simulink model "roach2_fengine"  (Simulink 7.9)
settings: Solver=ode45  StartTime=0.0  StopTime=10.0  RelTol=1e-3
undefined symbols (not defined in the file or in its folder): sfp
libraries: xbsIndex_r4 x3392, casper_library_sources x16, xps_library x13, casper_library_misc x6
(part 1 of 38)
settings Model: Creator=mdexter  LastModifiedBy=peter
settings DebuggingCC: FcnCallInpInsideContextMsg=Use local settings
settings GRTTargetCC: CombineOutputUpdateFcns=off  GRTInterface=on

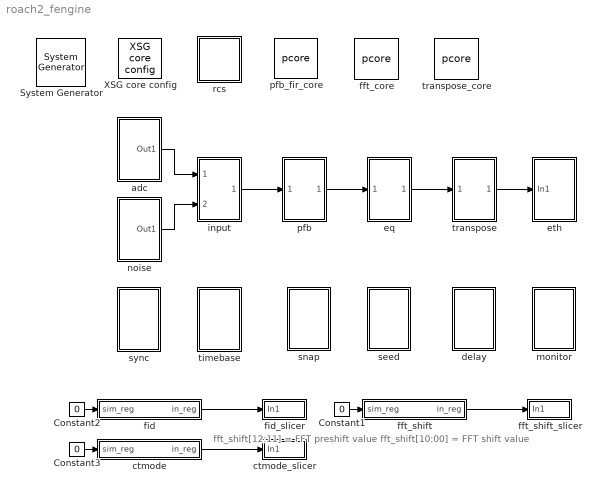
== system roach2_fengine ==
  note: fft_shift[12:11] = FFT preshift value
      | fft_shift[10:00] = FFT shift value
  " System Generator" : xbsIndex_r4/ System Generator  Tag=genX  xilinxfamily=virtex6  part=xc6vsx475t  speed=-1  package=ff1759  synthesis_tool=XST  clock_wrapper=Clock Enables  directory=./roach2_fengine/sysgen  proj_type=Project Navigator  Synth_file=XST Defaults  Impl_file=ISE Defaults  testbench=off  simulink_period=1  sysclk_period=4.1684  dcm_input_clock_period=100  incr_netlist=off  trim_vbits=Everywhere in SubSystem  dbl_ovrd=According to Block Masks  core_generation=According to Block Masks  run_coregen=off  deprecated_control=off  eval_field=0  block_type=sysgen
      settings: block_version=10.1.3
  Constant1 : Constant  Value=0
  Constant2 : Constant  Value=0
  Constant3 : Constant  Value=0
  XSG core config : xps_library/XSG core config  Tag=xps:xsg  hw_sys=ROACH2:sx475t  clk_src=adc0_clk  ROACH_clk_src=adc0_clk  ROACH2_clk_src=adc0_clk  clk_rate=200  sample_period=1  synthesis_tool=XST
  adc : Subsystem
  ctmode : Subsystem  Tag=xps:sw_reg  AncestorBlock=xps_library/software register  mask{numios=1, io_dir=From Processor, io_delay=0, sample_period=1, name1=reg, bitwidth1=32, arith_type1=Unsigned, bin_pt1=0}
      mask prompts: numios "Num I/Os"; io_dir "I/O direction"; io_delay "I/O delay"; sample_period "Sample period"; name1 "Name"; bitwidth1 "Bitwidth"; arith_type1 "Type"; bin_pt1 "Binary pt"
      mask init:
      | swreg_init(gcb);
      mask StyleString: edit,popup(To Processor|From Processor),edit,edit,edit,edit,popup(Unsigned|Signed  (2's comp)|Boolean),edit
      mask CallbackString:
      | t = timer('StartDelay', 0.1); t.TimerFcn = {@swreg_cb, gcb}; start(t);|a = get_param(gcb, 'MaskEnables');
      | if strcmp(get_param(gcb, 'io_dir'), 'To Processor'),
      | a(4) = {'off'};
      | else
      | a(4) = {'on'};
      | end
      | set_param(gcb, 'MaskEnables', a);
      | |||||a = get_param(gcb, 'MaskEnables');
      | if strcmp(get_param(gcb, 'arith_type1'), 'Boolean'),
      | set_param(gcb, 'bitwidth1', '1');
      | set_param(gcb, 'bin_pt1', '0');
      | a(6) = {'off'};
      | a(8) = {'off'};
      | else
      | a(6) = {'on'};
      | a(8) = {'on'};
      | end
      | set_param(gcb, 'MaskEnables', a);
      | |
      mask TabNameString: Setup,Setup,Setup,Setup,IO1,IO1,IO1,IO1
  ctmode_slicer : Subsystem
  delay : Subsystem
  eq : Subsystem
  eth : Subsystem
  fft_core : xps_library/pcore  Tag=xps:pcore  pcore_path=fft_1024ch_core/fft_1024ch_core.ngc
  fft_shift : Subsystem  Tag=xps:sw_reg  AncestorBlock=xps_library/software register  mask{numios=1, io_dir=From Processor, io_delay=0, sample_period=1, name1=reg, bitwidth1=32, arith_type1=Unsigned, bin_pt1=0}
      mask prompts: numios "Num I/Os"; io_dir "I/O direction"; io_delay "I/O delay"; sample_period "Sample period"; name1 "Name"; bitwidth1 "Bitwidth"; arith_type1 "Type"; bin_pt1 "Binary pt"
      mask init:
      | swreg_init(gcb);
      mask StyleString: edit,popup(To Processor|From Processor),edit,edit,edit,edit,popup(Unsigned|Signed  (2's comp)|Boolean),edit
      mask CallbackString:
      | t = timer('StartDelay', 0.1); t.TimerFcn = {@swreg_cb, gcb}; start(t);|a = get_param(gcb, 'MaskEnables');
      | if strcmp(get_param(gcb, 'io_dir'), 'To Processor'),
      | a(4) = {'off'};
      | else
      | a(4) = {'on'};
      | end
      | set_param(gcb, 'MaskEnables', a);
      | |||||a = get_param(gcb, 'MaskEnables');
      | if strcmp(get_param(gcb, 'arith_type1'), 'Boolean'),
      | set_param(gcb, 'bitwidth1', '1');
      | set_param(gcb, 'bin_pt1', '0');
      | a(6) = {'off'};
      | a(8) = {'off'};
      | else
      | a(6) = {'on'};
      | a(8) = {'on'};
      | end
      | set_param(gcb, 'MaskEnables', a);
      | |
      mask TabNameString: Setup,Setup,Setup,Setup,IO1,IO1,IO1,IO1
  fft_shift_slicer : Subsystem
  fid : Subsystem  Tag=xps:sw_reg  AncestorBlock=xps_library/software register  mask{numios=1, io_dir=From Processor, io_delay=0, sample_period=1, name1=reg, bitwidth1=32, arith_type1=Unsigned, bin_pt1=0}
      mask prompts: numios "Num I/Os"; io_dir "I/O direction"; io_delay "I/O delay"; sample_period "Sample period"; name1 "Name"; bitwidth1 "Bitwidth"; arith_type1 "Type"; bin_pt1 "Binary pt"
      mask init:
      | swreg_init(gcb);
      mask StyleString: edit,popup(To Processor|From Processor),edit,edit,edit,edit,popup(Unsigned|Signed  (2's comp)|Boolean),edit
      mask CallbackString:
      | t = timer('StartDelay', 0.1); t.TimerFcn = {@swreg_cb, gcb}; start(t);|a = get_param(gcb, 'MaskEnables');
      | if strcmp(get_param(gcb, 'io_dir'), 'To Processor'),
      | a(4) = {'off'};
      | else
      | a(4) = {'on'};
      | end
      | set_param(gcb, 'MaskEnables', a);
      | |||||a = get_param(gcb, 'MaskEnables');
      | if strcmp(get_param(gcb, 'arith_type1'), 'Boolean'),
      | set_param(gcb, 'bitwidth1', '1');
      | set_param(gcb, 'bin_pt1', '0');
      | a(6) = {'off'};
      | a(8) = {'off'};
      | else
      | a(6) = {'on'};
      | a(8) = {'on'};
      | end
      | set_param(gcb, 'MaskEnables', a);
      | |
      mask TabNameString: Setup,Setup,Setup,Setup,IO1,IO1,IO1,IO1
  fid_slicer : Subsystem
  input : Subsystem
  monitor : Subsystem
  noise : Subsystem
  pfb : Subsystem
  pfb_fir_core : xps_library/pcore  Tag=xps:pcore  pcore_path=pfb_fir_1024ch_core/pfb_fir_1024ch_core.ngc
  rcs : Subsystem  AncestorBlock=casper_library_misc/rcs  mask(rcs){app_src=git, lib_src=git, user_val=0}
      mask prompts: app_src "Application revision info to capture"; lib_src "CASPER libraries revision info to capture"; user_val "User specified value"
      mask help:
      | Embeds revision control information for the user design and libraries.
      | On failure to locate specified revision information, falls back to
      | timestamps (current time for libraries).
      | A user specified value is also stored.
      mask init:
      | rcs_init(gcb, ...
      |     'app_src', app_src, ...
      |     'lib_src', lib_src);
      mask StyleString: popup(timestamp|git|svn),popup(timestamp|git|svn),edit
  seed : Subsystem
  snap : Subsystem
  sync : Subsystem
  timebase : Subsystem
  transpose : Subsystem
  transpose_core : xps_library/pcore  Tag=xps:pcore  pcore_path=transpose_core/transpose_core.ngc
  wires:
  input/1(Out1) -> pfb/1(In1)
  pfb/1(Out1) -> eq/1(In1)
  eq/1(Out2) -> transpose/1(In1)
  adc/1(Out1) -> input/1(In1)
  noise/1(Out1) -> input/2(In2)
  transpose/1(Out1) -> eth/1(In1)
  fft_shift/1(in_reg) -> fft_shift_slicer/1(In1)
  Constant1/1 -> fft_shift/1(sim_reg)
  fid/1(in_reg) -> fid_slicer/1(In1)
  Constant2/1 -> fid/1(sim_reg)
  ctmode/1(in_reg) -> ctmode_slicer/1(In1)
  Constant3/1 -> ctmode/1(sim_reg)

== system roach2_fengine/adc ==
  Bus Creator : BusCreator  Inputs=32
  Constant{3..34} (32 alike) : Constant  Value=0
  adc16x250-8 : Subsystem  Tag=xps:adc16  AncestorBlock=xps_library/ADCs/adc16x250-8  mask(ADC16){board_count=2, roach2_rev=2, zdok_rev=2}
      mask prompts: board_count "Number of ADC16 Boards"; roach2_rev "ROACH2 Revision"; zdok_rev "ZDOK Pinout Revision"
      mask help: 16-input ADC. When using one ADC16 board, it must be in ZDOK0.
      mask init:
      | % Only board_count is needed by init function
      | adc16_init(gcb, ...
      |     'board_count', board_count);
      settings: AttributesFormatString=r2 rev%<roach2_rev>\nzdok rev%<zdok_rev>
      mask Help:
      | This is the CASPER "yellow block" for the CASPER ADC16 board.  Each ADC
      | board has four ADC chips.  Each ADC chip accepts four inputs.  The four
      | 8-bit outputs from each ADC chip are concatenated into a 32-bit signal.
      mask StyleString: popup(1|2),popup(1|2),popup(1|2)
  Out1 : Outport
  wires:
  Constant3/1 -> adc16x250-8/1(a1_sim)
  Constant4/1 -> adc16x250-8/2(a2_sim)
  Constant5/1 -> adc16x250-8/3(a3_sim)
  Constant6/1 -> adc16x250-8/4(a4_sim)
  Constant7/1 -> adc16x250-8/5(b1_sim)
  Constant8/1 -> adc16x250-8/6(b2_sim)
  Constant9/1 -> adc16x250-8/7(b3_sim)
  Constant33/1 -> adc16x250-8/8(b4_sim)
  Constant34/1 -> adc16x250-8/9(c1_sim)
  Constant10/1 -> adc16x250-8/10(c2_sim)
  Constant11/1 -> adc16x250-8/11(c3_sim)
  Constant12/1 -> adc16x250-8/12(c4_sim)
  Constant13/1 -> adc16x250-8/13(d1_sim)
  Constant14/1 -> adc16x250-8/14(d2_sim)
  Constant15/1 -> adc16x250-8/15(d3_sim)
  Constant17/1 -> adc16x250-8/16(d4_sim)
  Constant16/1 -> adc16x250-8/17(e1_sim)
  Constant25/1 -> adc16x250-8/18(e2_sim)
  Constant26/1 -> adc16x250-8/19(e3_sim)
  Constant27/1 -> adc16x250-8/20(e4_sim)
  Constant28/1 -> adc16x250-8/21(f1_sim)
  Constant29/1 -> adc16x250-8/22(f2_sim)
  Constant30/1 -> adc16x250-8/23(f3_sim)
  Constant31/1 -> adc16x250-8/24(f4_sim)
  Constant32/1 -> adc16x250-8/25(g1_sim)
  Constant18/1 -> adc16x250-8/26(g2_sim)
  Constant19/1 -> adc16x250-8/27(g3_sim)
  Constant20/1 -> adc16x250-8/28(g4_sim)
  Constant21/1 -> adc16x250-8/29(h1_sim)
  Constant22/1 -> adc16x250-8/30(h2_sim)
  Constant23/1 -> adc16x250-8/31(h3_sim)
  Constant24/1 -> adc16x250-8/32(h4_sim)
  Bus Creator/1 -> Out1/1
  adc16x250-8/1(a1) -> Bus Creator/1
  adc16x250-8/2(a2) -> Bus Creator/2
  adc16x250-8/3(a3) -> Bus Creator/3
  adc16x250-8/4(a4) -> Bus Creator/4
  adc16x250-8/5(b1) -> Bus Creator/5
  adc16x250-8/6(b2) -> Bus Creator/6
  adc16x250-8/7(b3) -> Bus Creator/7
  adc16x250-8/8(b4) -> Bus Creator/8
  adc16x250-8/9(c1) -> Bus Creator/9
  adc16x250-8/10(c2) -> Bus Creator/10
  adc16x250-8/11(c3) -> Bus Creator/11
  adc16x250-8/12(c4) -> Bus Creator/12
  adc16x250-8/13(d1) -> Bus Creator/13
  adc16x250-8/14(d2) -> Bus Creator/14
  adc16x250-8/15(d3) -> Bus Creator/15
  adc16x250-8/16(d4) -> Bus Creator/16
  adc16x250-8/17(e1) -> Bus Creator/17
  adc16x250-8/18(e2) -> Bus Creator/18
  adc16x250-8/19(e3) -> Bus Creator/19
  adc16x250-8/20(e4) -> Bus Creator/20
  adc16x250-8/21(f1) -> Bus Creator/21
  adc16x250-8/22(f2) -> Bus Creator/22
  adc16x250-8/23(f3) -> Bus Creator/23
  adc16x250-8/24(f4) -> Bus Creator/24
  adc16x250-8/25(g1) -> Bus Creator/25
  adc16x250-8/26(g2) -> Bus Creator/26
  adc16x250-8/27(g3) -> Bus Creator/27
  adc16x250-8/28(g4) -> Bus Creator/28
  adc16x250-8/29(h1) -> Bus Creator/29
  adc16x250-8/30(h2) -> Bus Creator/30
  adc16x250-8/31(h3) -> Bus Creator/31
  adc16x250-8/32(h4) -> Bus Creator/32

== system roach2_fengine/ctmode ==
  sim_reg : Inport
  io_delay : xbsIndex_r4/Delay  rst=off  infoeditControl=Selection of Reset will increase slice count due to use of real FFs and instead of SRLs  en=off  latency=0  dbl_ovrd=off  reg_retiming=on  block_type=delay
  reint1 : xbsIndex_r4/Reinterpret  force_arith_type=on  arith_type=Unsigned  force_bin_pt=on  bin_pt=0  block_type=reinterpret
  roach2_fengine_ctmode_user_data_out : xbsIndex_r4/Gateway In  gui_display_data_type=Fixed-point  arith_type=Unsigned  n_bits=32  bin_pt=0  preci_type=Single  exp_width=8  frac_width=24  quantization="Round  (unbiased: +/- Inf)"  overflow=Saturate  period=1  dbl_ovrd=off  timing_constraint=None  locs_specified=off  LOCs={}  UseAsADC=off  ADCChannel='1'  inherit_from_input=off  hdl_port=on  block_type=gatewayin
  slice_reg : xbsIndex_r4/Slice  nbits=32  boolean_output=off  mode=Lower Bit Location + Width  bit1=0  base1=MSB of Input  bit0=0  base0=LSB of Input  dbl_ovrd=off  block_type=slice
  in_reg : Outport
  wires:
  roach2_fengine_ctmode_user_data_out/1 -> io_delay/1
  sim_reg/1 -> roach2_fengine_ctmode_user_data_out/1
  io_delay/1 -> slice_reg/1
  slice_reg/1 -> reint1/1
  reint1/1 -> in_reg/1

== system roach2_fengine/ctmode_slicer ==
  In1 : Inport
  Delay8 : xbsIndex_r4/Delay  rst=off  infoeditControl=Selection of Reset will increase slice count due to use of real FFs and instead of SRLs  en=off  latency=2  dbl_ovrd=off  reg_retiming=on  block_type=delay
      settings: xl_area=[0, 0, 0, 0, 0, 0, 0]
  b1_b0 : xbsIndex_r4/Slice  nbits=2  boolean_output=off  mode=Lower Bit Location + Width  bit1=0  base1=MSB of Input  bit0=0  base0=LSB of Input  dbl_ovrd=off  block_type=slice
      settings: block_version=10.1.3
  tag scope: ctmode=global
  wires:
  Delay8/1 -> b1_b0/1
  b1_b0/1 -> [ctmode]
  In1/1 -> Delay8/1

== system roach2_fengine/delay ==
  0 : Subsystem  Tag=xps:sw_reg  AncestorBlock=xps_library/software register  mask{numios=1, io_dir=From Processor, io_delay=0, sample_period=1, name1=reg, bitwidth1=32, arith_type1=Unsigned, bin_pt1=0}
      mask prompts: numios "Num I/Os"; io_dir "I/O direction"; io_delay "I/O delay"; sample_period "Sample period"; name1 "Name"; bitwidth1 "Bitwidth"; arith_type1 "Type"; bin_pt1 "Binary pt"
      mask init:
      | swreg_init(gcb);
      mask StyleString: edit,popup(To Processor|From Processor),edit,edit,edit,edit,popup(Unsigned|Signed  (2's comp)|Boolean),edit
      mask CallbackString:
      | t = timer('StartDelay', 0.1); t.TimerFcn = {@swreg_cb, gcb}; start(t);|a = get_param(gcb, 'MaskEnables');
      | if strcmp(get_param(gcb, 'io_dir'), 'To Processor'),
      | a(4) = {'off'};
      | else
      | a(4) = {'on'};
      | end
      | set_param(gcb, 'MaskEnables', a);
      | |||||a = get_param(gcb, 'MaskEnables');
      | if strcmp(get_param(gcb, 'arith_type1'), 'Boolean'),
      | set_param(gcb, 'bitwidth1', '1');
      | set_param(gcb, 'bin_pt1', '0');
      | a(6) = {'off'};
      | a(8) = {'off'};
      | else
      | a(6) = {'on'};
      | a(8) = {'on'};
      | end
      | set_param(gcb, 'MaskEnables', a);
      | |
      mask TabNameString: Setup,Setup,Setup,Setup,IO1,IO1,IO1,IO1
  1 : Subsystem  Tag=xps:sw_reg  AncestorBlock=xps_library/software register  mask{numios=1, io_dir=From Processor, io_delay=0, sample_period=1, name1=reg, bitwidth1=32, arith_type1=Unsigned, bin_pt1=0}
      mask prompts: numios "Num I/Os"; io_dir "I/O direction"; io_delay "I/O delay"; sample_period "Sample period"; name1 "Name"; bitwidth1 "Bitwidth"; arith_type1 "Type"; bin_pt1 "Binary pt"
      mask init:
      | swreg_init(gcb);
      mask StyleString: edit,popup(To Processor|From Processor),edit,edit,edit,edit,popup(Unsigned|Signed  (2's comp)|Boolean),edit
      mask CallbackString:
      | t = timer('StartDelay', 0.1); t.TimerFcn = {@swreg_cb, gcb}; start(t);|a = get_param(gcb, 'MaskEnables');
      | if strcmp(get_param(gcb, 'io_dir'), 'To Processor'),
      | a(4) = {'off'};
      | else
      | a(4) = {'on'};
      | end
      | set_param(gcb, 'MaskEnables', a);
      | |||||a = get_param(gcb, 'MaskEnables');
      | if strcmp(get_param(gcb, 'arith_type1'), 'Boolean'),
      | set_param(gcb, 'bitwidth1', '1');
      | set_param(gcb, 'bin_pt1', '0');
      | a(6) = {'off'};
      | a(8) = {'off'};
      | else
      | a(6) = {'on'};
      | a(8) = {'on'};
      | end
      | set_param(gcb, 'MaskEnables', a);
      | |
      mask TabNameString: Setup,Setup,Setup,Setup,IO1,IO1,IO1,IO1
  2 : Subsystem  Tag=xps:sw_reg  AncestorBlock=xps_library/software register  mask{numios=1, io_dir=From Processor, io_delay=0, sample_period=1, name1=reg, bitwidth1=32, arith_type1=Unsigned, bin_pt1=0}
      mask prompts: numios "Num I/Os"; io_dir "I/O direction"; io_delay "I/O delay"; sample_period "Sample period"; name1 "Name"; bitwidth1 "Bitwidth"; arith_type1 "Type"; bin_pt1 "Binary pt"
      mask init:
      | swreg_init(gcb);
      mask StyleString: edit,popup(To Processor|From Processor),edit,edit,edit,edit,popup(Unsigned|Signed  (2's comp)|Boolean),edit
      mask CallbackString:
      | t = timer('StartDelay', 0.1); t.TimerFcn = {@swreg_cb, gcb}; start(t);|a = get_param(gcb, 'MaskEnables');
      | if strcmp(get_param(gcb, 'io_dir'), 'To Processor'),
      | a(4) = {'off'};
      | else
      | a(4) = {'on'};
      | end
      | set_param(gcb, 'MaskEnables', a);
      | |||||a = get_param(gcb, 'MaskEnables');
      | if strcmp(get_param(gcb, 'arith_type1'), 'Boolean'),
      | set_param(gcb, 'bitwidth1', '1');
      | set_param(gcb, 'bin_pt1', '0');
      | a(6) = {'off'};
      | a(8) = {'off'};
      | else
      | a(6) = {'on'};
      | a(8) = {'on'};
      | end
      | set_param(gcb, 'MaskEnables', a);
      | |
      mask TabNameString: Setup,Setup,Setup,Setup,IO1,IO1,IO1,IO1
  3 : Subsystem  Tag=xps:sw_reg  AncestorBlock=xps_library/software register  mask{numios=1, io_dir=From Processor, io_delay=0, sample_period=1, name1=reg, bitwidth1=32, arith_type1=Unsigned, bin_pt1=0}
      mask prompts: numios "Num I/Os"; io_dir "I/O direction"; io_delay "I/O delay"; sample_period "Sample period"; name1 "Name"; bitwidth1 "Bitwidth"; arith_type1 "Type"; bin_pt1 "Binary pt"
      mask init:
      | swreg_init(gcb);
      mask StyleString: edit,popup(To Processor|From Processor),edit,edit,edit,edit,popup(Unsigned|Signed  (2's comp)|Boolean),edit
      mask CallbackString:
      | t = timer('StartDelay', 0.1); t.TimerFcn = {@swreg_cb, gcb}; start(t);|a = get_param(gcb, 'MaskEnables');
      | if strcmp(get_param(gcb, 'io_dir'), 'To Processor'),
      | a(4) = {'off'};
      | else
      | a(4) = {'on'};
      | end
      | set_param(gcb, 'MaskEnables', a);
      | |||||a = get_param(gcb, 'MaskEnables');
      | if strcmp(get_param(gcb, 'arith_type1'), 'Boolean'),
      | set_param(gcb, 'bitwidth1', '1');
      | set_param(gcb, 'bin_pt1', '0');
      | a(6) = {'off'};
      | a(8) = {'off'};
      | else
      | a(6) = {'on'};
      | a(8) = {'on'};
      | end
      | set_param(gcb, 'MaskEnables', a);
      | |
      mask TabNameString: Setup,Setup,Setup,Setup,IO1,IO1,IO1,IO1
  4 : Subsystem  Tag=xps:sw_reg  AncestorBlock=xps_library/software register  mask{numios=1, io_dir=From Processor, io_delay=0, sample_period=1, name1=reg, bitwidth1=32, arith_type1=Unsigned, bin_pt1=0}
      mask prompts: numios "Num I/Os"; io_dir "I/O direction"; io_delay "I/O delay"; sample_period "Sample period"; name1 "Name"; bitwidth1 "Bitwidth"; arith_type1 "Type"; bin_pt1 "Binary pt"
      mask init:
      | swreg_init(gcb);
      mask StyleString: edit,popup(To Processor|From Processor),edit,edit,edit,edit,popup(Unsigned|Signed  (2's comp)|Boolean),edit
      mask CallbackString:
      | t = timer('StartDelay', 0.1); t.TimerFcn = {@swreg_cb, gcb}; start(t);|a = get_param(gcb, 'MaskEnables');
      | if strcmp(get_param(gcb, 'io_dir'), 'To Processor'),
      | a(4) = {'off'};
      | else
      | a(4) = {'on'};
      | end
      | set_param(gcb, 'MaskEnables', a);
      | |||||a = get_param(gcb, 'MaskEnables');
      | if strcmp(get_param(gcb, 'arith_type1'), 'Boolean'),
      | set_param(gcb, 'bitwidth1', '1');
      | set_param(gcb, 'bin_pt1', '0');
      | a(6) = {'off'};
      | a(8) = {'off'};
      | else
      | a(6) = {'on'};
      | a(8) = {'on'};
      | end
      | set_param(gcb, 'MaskEnables', a);
      | |
      mask TabNameString: Setup,Setup,Setup,Setup,IO1,IO1,IO1,IO1
  5 : Subsystem  Tag=xps:sw_reg  AncestorBlock=xps_library/software register  mask{numios=1, io_dir=From Processor, io_delay=0, sample_period=1, name1=reg, bitwidth1=32, arith_type1=Unsigned, bin_pt1=0}
      mask prompts: numios "Num I/Os"; io_dir "I/O direction"; io_delay "I/O delay"; sample_period "Sample period"; name1 "Name"; bitwidth1 "Bitwidth"; arith_type1 "Type"; bin_pt1 "Binary pt"
      mask init:
      | swreg_init(gcb);
      mask StyleString: edit,popup(To Processor|From Processor),edit,edit,edit,edit,popup(Unsigned|Signed  (2's comp)|Boolean),edit
      mask CallbackString:
      | t = timer('StartDelay', 0.1); t.TimerFcn = {@swreg_cb, gcb}; start(t);|a = get_param(gcb, 'MaskEnables');
      | if strcmp(get_param(gcb, 'io_dir'), 'To Processor'),
      | a(4) = {'off'};
      | else
      | a(4) = {'on'};
      | end
      | set_param(gcb, 'MaskEnables', a);
      | |||||a = get_param(gcb, 'MaskEnables');
      | if strcmp(get_param(gcb, 'arith_type1'), 'Boolean'),
      | set_param(gcb, 'bitwidth1', '1');
      | set_param(gcb, 'bin_pt1', '0');
      | a(6) = {'off'};
      | a(8) = {'off'};
      | else
      | a(6) = {'on'};
      | a(8) = {'on'};
      | end
      | set_param(gcb, 'MaskEnables', a);
      | |
      mask TabNameString: Setup,Setup,Setup,Setup,IO1,IO1,IO1,IO1
  6 : Subsystem  Tag=xps:sw_reg  AncestorBlock=xps_library/software register  mask{numios=1, io_dir=From Processor, io_delay=0, sample_period=1, name1=reg, bitwidth1=32, arith_type1=Unsigned, bin_pt1=0}
      mask prompts: numios "Num I/Os"; io_dir "I/O direction"; io_delay "I/O delay"; sample_period "Sample period"; name1 "Name"; bitwidth1 "Bitwidth"; arith_type1 "Type"; bin_pt1 "Binary pt"
      mask init:
      | swreg_init(gcb);
      mask StyleString: edit,popup(To Processor|From Processor),edit,edit,edit,edit,popup(Unsigned|Signed  (2's comp)|Boolean),edit
      mask CallbackString:
      | t = timer('StartDelay', 0.1); t.TimerFcn = {@swreg_cb, gcb}; start(t);|a = get_param(gcb, 'MaskEnables');
      | if strcmp(get_param(gcb, 'io_dir'), 'To Processor'),
      | a(4) = {'off'};
      | else
      | a(4) = {'on'};
      | end
      | set_param(gcb, 'MaskEnables', a);
      | |||||a = get_param(gcb, 'MaskEnables');
      | if strcmp(get_param(gcb, 'arith_type1'), 'Boolean'),
      | set_param(gcb, 'bitwidth1', '1');
      | set_param(gcb, 'bin_pt1', '0');
      | a(6) = {'off'};
      | a(8) = {'off'};
      | else
      | a(6) = {'on'};
      | a(8) = {'on'};
      | end
      | set_param(gcb, 'MaskEnables', a);
      | |
      mask TabNameString: Setup,Setup,Setup,Setup,IO1,IO1,IO1,IO1
  7 : Subsystem  Tag=xps:sw_reg  AncestorBlock=xps_library/software register  mask{numios=1, io_dir=From Processor, io_delay=0, sample_period=1, name1=reg, bitwidth1=32, arith_type1=Unsigned, bin_pt1=0}
      mask prompts: numios "Num I/Os"; io_dir "I/O direction"; io_delay "I/O delay"; sample_period "Sample period"; name1 "Name"; bitwidth1 "Bitwidth"; arith_type1 "Type"; bin_pt1 "Binary pt"
      mask init:
      | swreg_init(gcb);
      mask StyleString: edit,popup(To Processor|From Processor),edit,edit,edit,edit,popup(Unsigned|Signed  (2's comp)|Boolean),edit
      mask CallbackString:
      | t = timer('StartDelay', 0.1); t.TimerFcn = {@swreg_cb, gcb}; start(t);|a = get_param(gcb, 'MaskEnables');
      | if strcmp(get_param(gcb, 'io_dir'), 'To Processor'),
      | a(4) = {'off'};
      | else
      | a(4) = {'on'};
      | end
      | set_param(gcb, 'MaskEnables', a);
      | |||||a = get_param(gcb, 'MaskEnables');
      | if strcmp(get_param(gcb, 'arith_type1'), 'Boolean'),
      | set_param(gcb, 'bitwidth1', '1');
      | set_param(gcb, 'bin_pt1', '0');
      | a(6) = {'off'};
      | a(8) = {'off'};
      | else
      | a(6) = {'on'};
      | a(8) = {'on'};
      | end
      | set_param(gcb, 'MaskEnables', a);
      | |
      mask TabNameString: Setup,Setup,Setup,Setup,IO1,IO1,IO1,IO1
  BitBasher : xbsIndex_r4/BitBasher  bitexpr=|  display_expr=off  sr_1=1  arith_type1=Unsigned  bin_pt1=0  sr_2=2  arith_type2=Unsigned  bin_pt2=0  sr_3=3  arith_type3=Unsigned  bin_pt3=0  sr_4=4  arith_type4=Unsigned  bin_pt4=0  dbl_ovrd=off  block_type=bitbasher
      | <!DOCTYPE HTML PUBLIC "-//W3C//DTD HTML 4.0//EN" "http://www.w3.org/TR/REC-html40/strict.dtd">
      | <html><head><meta name="qrichtext" content="1" /><style type="text/css">
      | p, li { white-space: pre-wrap; }
      | </style></head><body style=" font-family:'Sans'; font-size:10pt; font-weight:400; font-style:normal;">
      | <p style=" margin-top:12px; margin-bottom:12px; margin-left:0px; margin-right:0px; -qt-block-indent:0; text-indent:0px;"><span style=" font-family:'MS Shell Dlg'; font-size:8pt;">d={b,a}</span></p></body></html>
  BitBasher1 : xbsIndex_r4/BitBasher  bitexpr=|  display_expr=off  sr_1=1  arith_type1=Unsigned  bin_pt1=0  sr_2=2  arith_type2=Unsigned  bin_pt2=0  sr_3=3  arith_type3=Unsigned  bin_pt3=0  sr_4=4  arith_type4=Unsigned  bin_pt4=0  dbl_ovrd=off  block_type=bitbasher
      | <!DOCTYPE HTML PUBLIC "-//W3C//DTD HTML 4.0//EN" "http://www.w3.org/TR/REC-html40/strict.dtd">
      | <html><head><meta name="qrichtext" content="1" /><style type="text/css">
      | p, li { white-space: pre-wrap; }
      | </style></head><body style=" font-family:'Sans'; font-size:10pt; font-weight:400; font-style:normal;">
      | <p style=" margin-top:12px; margin-bottom:12px; margin-left:0px; margin-right:0px; -qt-block-indent:0; text-indent:0px;"><span style=" font-family:'MS Shell Dlg'; font-size:8pt;">d={b,a}</span></p></body></html>
  BitBasher2 : xbsIndex_r4/BitBasher  bitexpr=|  display_expr=off  sr_1=1  arith_type1=Unsigned  bin_pt1=0  sr_2=2  arith_type2=Unsigned  bin_pt2=0  sr_3=3  arith_type3=Unsigned  bin_pt3=0  sr_4=4  arith_type4=Unsigned  bin_pt4=0  dbl_ovrd=off  block_type=bitbasher
      | <!DOCTYPE HTML PUBLIC "-//W3C//DTD HTML 4.0//EN" "http://www.w3.org/TR/REC-html40/strict.dtd">
      | <html><head><meta name="qrichtext" content="1" /><style type="text/css">
      | p, li { white-space: pre-wrap; }
      | </style></head><body style=" font-family:'Sans'; font-size:10pt; font-weight:400; font-style:normal;">
      | <p style=" margin-top:12px; margin-bottom:12px; margin-left:0px; margin-right:0px; -qt-block-indent:0; text-indent:0px;"><span style=" font-family:'MS Shell Dlg'; font-size:8pt;">d={b,a}</span></p></body></html>
  BitBasher3 : xbsIndex_r4/BitBasher  bitexpr=|  display_expr=off  sr_1=1  arith_type1=Unsigned  bin_pt1=0  sr_2=2  arith_type2=Unsigned  bin_pt2=0  sr_3=3  arith_type3=Unsigned  bin_pt3=0  sr_4=4  arith_type4=Unsigned  bin_pt4=0  dbl_ovrd=off  block_type=bitbasher
      | <!DOCTYPE HTML PUBLIC "-//W3C//DTD HTML 4.0//EN" "http://www.w3.org/TR/REC-html40/strict.dtd">
      | <html><head><meta name="qrichtext" content="1" /><style type="text/css">
      | p, li { white-space: pre-wrap; }
      | </style></head><body style=" font-family:'Sans'; font-size:10pt; font-weight:400; font-style:normal;">
      | <p style=" margin-top:12px; margin-bottom:12px; margin-left:0px; margin-right:0px; -qt-block-indent:0; text-indent:0px;"><span style=" font-family:'MS Shell Dlg'; font-size:8pt;">d={b,a}</span></p></body></html>
  Constant{,1..7} (8 alike) : Constant  Value=0
  tag scope: delay0=global, delay1=global, delay2=global, delay3=global
  wires:
  0/1(in_reg) -> BitBasher/1
  Constant/1 -> 0/1(sim_reg)
  1/1(in_reg) -> BitBasher/2
  Constant1/1 -> 1/1(sim_reg)
  2/1(in_reg) -> BitBasher1/1
  Constant2/1 -> 2/1(sim_reg)
  3/1(in_reg) -> BitBasher1/2
  Constant3/1 -> 3/1(sim_reg)
  4/1(in_reg) -> BitBasher2/1
  Constant4/1 -> 4/1(sim_reg)
  5/1(in_reg) -> BitBasher2/2
  Constant5/1 -> 5/1(sim_reg)
  6/1(in_reg) -> BitBasher3/1
  Constant6/1 -> 6/1(sim_reg)
  7/1(in_reg) -> BitBasher3/2
  Constant7/1 -> 7/1(sim_reg)
  BitBasher/1 -> [delay0]
  BitBasher1/1 -> [delay1]
  BitBasher2/1 -> [delay2]
  BitBasher3/1 -> [delay3]

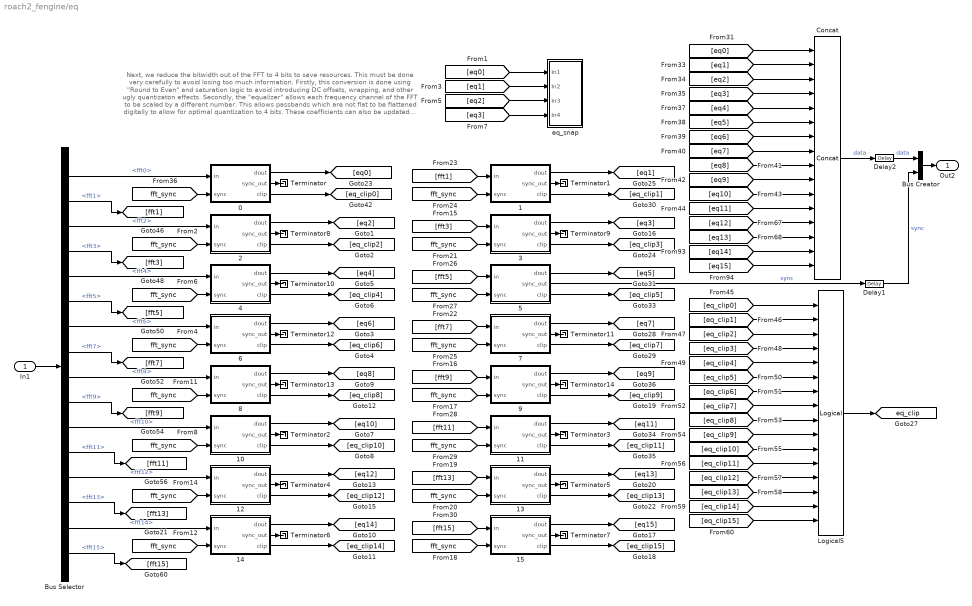
== system roach2_fengine/eq ==
  note: Next, we reduce the bitwidth out of the FFT to 4 bits to save resources.
      | This must be done very carefully to avoid losing too much information.
      | Firstly, this conversion is done using "Round to Even" and saturation
      | logic to avoid introducing DC offsets, wrapping, and other ugly quantizaton
      | effects.  Secondly, the "equalizer" allows each frequency channel of the
      | FFT to be scaled by a different number.  This allows passbands which are
      | not flat to be flattened digitally to allow for optimal quantization to 4 bits.
      | These coefficients can also be updated dynamically for gain control.
      | Coefficients can be dynamically written into the "equalizer" by
      | specifying the channel ("coeff_addr") and the value of the
      | coefficient ("coeff"), and then using "coeff_en" to write the
      | new coefficient into the equalization table.
  In1 : Inport
  0 : Subsystem  mask{nchan=11}
      mask prompts: nchan "Number of fft channels (same as fft prompt) (2^?)"
  1 : Subsystem  mask{nchan=11}
      mask prompts: nchan "Number of fft channels (same as fft prompt) (2^?)"
  10 : Subsystem  mask{nchan=11}
      mask prompts: nchan "Number of fft channels (same as fft prompt) (2^?)"
  11 : Subsystem  mask{nchan=11}
      mask prompts: nchan "Number of fft channels (same as fft prompt) (2^?)"
  12 : Subsystem  mask{nchan=11}
      mask prompts: nchan "Number of fft channels (same as fft prompt) (2^?)"
  13 : Subsystem  mask{nchan=11}
      mask prompts: nchan "Number of fft channels (same as fft prompt) (2^?)"
  14 : Subsystem  mask{nchan=11}
      mask prompts: nchan "Number of fft channels (same as fft prompt) (2^?)"
  15 : Subsystem  mask{nchan=11}
      mask prompts: nchan "Number of fft channels (same as fft prompt) (2^?)"
  2 : Subsystem  mask{nchan=11}
      mask prompts: nchan "Number of fft channels (same as fft prompt) (2^?)"
  3 : Subsystem  mask{nchan=11}
      mask prompts: nchan "Number of fft channels (same as fft prompt) (2^?)"
  4 : Subsystem  mask{nchan=11}
      mask prompts: nchan "Number of fft channels (same as fft prompt) (2^?)"
  5 : Subsystem  mask{nchan=11}
      mask prompts: nchan "Number of fft channels (same as fft prompt) (2^?)"
  6 : Subsystem  mask{nchan=11}
      mask prompts: nchan "Number of fft channels (same as fft prompt) (2^?)"
  7 : Subsystem  mask{nchan=11}
      mask prompts: nchan "Number of fft channels (same as fft prompt) (2^?)"
  8 : Subsystem  mask{nchan=11}
      mask prompts: nchan "Number of fft channels (same as fft prompt) (2^?)"
  9 : Subsystem  mask{nchan=11}
      mask prompts: nchan "Number of fft channels (same as fft prompt) (2^?)"
  Bus Creator : BusCreator  Inputs=2
  Bus Selector : BusSelector  OutputSignals=fft0,fft1,fft2,fft3,fft4,fft5,fft6,fft7,fft8,fft9,fft10,fft11,fft12,fft13,fft14,fft15
  Concat : xbsIndex_r4/Concat  num_inputs=16  dbl_ovrd=off  block_type=concat
  Delay1 : xbsIndex_r4/Delay  rst=off  infoeditControl=Selection of Reset will increase slice count due to use of real FFs and instead of SRLs  en=off  latency=1  dbl_ovrd=off  reg_retiming=off  block_type=delay
  Delay2 : xbsIndex_r4/Delay  rst=off  infoeditControl=Selection of Reset will increase slice count due to use of real FFs and instead of SRLs  en=off  latency=1  dbl_ovrd=off  reg_retiming=off  block_type=delay
  Logical5 : xbsIndex_r4/Logical  logical_function=OR  inputs=16  en=off  latency=1  precision=Full  arith_type=Unsigned  n_bits=8  bin_pt=2  align_bp=on  dbl_ovrd=off  block_type=logical
      settings: xl_area=[0, 0, 0, 0, 0, 0, 0]
  Terminator{,1..14} (15 alike) : Terminator
  eq_snap : Subsystem
  Out2 : Outport
  tag scope: eq_clip=global
  wires:
  0/1(dout) -> [eq0]
  0/3(clip) -> [eq_clip0]
  Logical5/1 -> [eq_clip]
  [fft_sync] -> 0/2(sync)
  Delay2/1 -> Bus Creator/1  "data"
  Bus Creator/1 -> Out2/1
  2/1(dout) -> [eq2]
  2/3(clip) -> [eq_clip2]
  [fft_sync] -> 2/2(sync)
  4/1(dout) -> [eq4]
  Bus Selector/5 -> 4/1(in)  "<fft4>"
  4/3(clip) -> [eq_clip4]
  [fft_sync] -> 4/2(sync)
  6/1(dout) -> [eq6]
  Bus Selector/7 -> 6/1(in)  "<fft6>"
  6/3(clip) -> [eq_clip6]
  [fft_sync] -> 6/2(sync)
  8/1(dout) -> [eq8]
  Bus Selector/9 -> 8/1(in)  "<fft8>"
  8/3(clip) -> [eq_clip8]
  [fft_sync] -> 8/2(sync)
  10/1(dout) -> [eq10]
  Bus Selector/11 -> 10/1(in)  "<fft10>"
  10/3(clip) -> [eq_clip10]
  [fft_sync] -> 10/2(sync)
  12/1(dout) -> [eq12]
  Bus Selector/13 -> 12/1(in)  "<fft12>"
  12/3(clip) -> [eq_clip12]
  [fft_sync] -> 12/2(sync)
  14/1(dout) -> [eq14]
  Bus Selector/15 -> 14/1(in)  "<fft14>"
  14/3(clip) -> [eq_clip14]
  [fft_sync] -> 14/2(sync)
  1/1(dout) -> [eq1]
  1/3(clip) -> [eq_clip1]
  [fft_sync] -> 1/2(sync)
  3/1(dout) -> [eq3]
  [fft3] -> 3/1(in)
  3/3(clip) -> [eq_clip3]
  [fft_sync] -> 3/2(sync)
  5/1(dout) -> [eq5]
  [fft5] -> 5/1(in)
  5/3(clip) -> [eq_clip5]
  [fft_sync] -> 5/2(sync)
  7/1(dout) -> [eq7]
  [fft7] -> 7/1(in)
  7/3(clip) -> [eq_clip7]
  [fft_sync] -> 7/2(sync)
  9/1(dout) -> [eq9]
  [fft9] -> 9/1(in)
  9/3(clip) -> [eq_clip9]
  [fft_sync] -> 9/2(sync)
  11/1(dout) -> [eq11]
  [fft11] -> 11/1(in)
  11/3(clip) -> [eq_clip11]
  [fft_sync] -> 11/2(sync)
  13/1(dout) -> [eq13]
  [fft13] -> 13/1(in)
  13/3(clip) -> [eq_clip13]
  [fft_sync] -> 13/2(sync)
  15/1(dout) -> [eq15]
  [fft15] -> 15/1(in)
  15/3(clip) -> [eq_clip15]
  [fft_sync] -> 15/2(sync)
  [eq0] -> Concat/1
  [eq1] -> Concat/2
  [eq2] -> Concat/3
  [eq3] -> Concat/4
  [eq4] -> Concat/5
  [eq5] -> Concat/6
  [eq6] -> Concat/7
  [eq7] -> Concat/8
  [eq8] -> Concat/9
  [eq9] -> Concat/10
  [eq10] -> Concat/11
  [eq11] -> Concat/12
  [eq12] -> Concat/13
  [eq13] -> Concat/14
  [eq14] -> Concat/15
  [eq15] -> Concat/16
  [eq_clip0] -> Logical5/1
  [eq_clip1] -> Logical5/2
  [eq_clip2] -> Logical5/3
  [eq_clip3] -> Logical5/4
  [eq_clip4] -> Logical5/5
  [eq_clip5] -> Logical5/6
  [eq_clip6] -> Logical5/7
  [eq_clip7] -> Logical5/8
  [eq_clip8] -> Logical5/9
  [eq_clip9] -> Logical5/10
  [eq_clip10] -> Logical5/11
  [eq_clip11] -> Logical5/12
  [eq_clip12] -> Logical5/13
  [eq_clip13] -> Logical5/14
  [eq_clip14] -> Logical5/15
  [eq_clip15] -> Logical5/16
  In1/1 -> Bus Selector/1
  Bus Selector/1 -> 0/1(in)  "<fft0>"
  Bus Selector/3 -> 2/1(in)  "<fft2>"
  [fft1] -> 1/1(in)
  Bus Selector/16 -> [fft15]  "<fft15>"
  Bus Selector/14 -> [fft13]  "<fft13>"
  Bus Selector/12 -> [fft11]  "<fft11>"
  Bus Selector/10 -> [fft9]  "<fft9>"
  Bus Selector/8 -> [fft7]  "<fft7>"
  Bus Selector/6 -> [fft5]  "<fft5>"
  Bus Selector/4 -> [fft3]  "<fft3>"
  Bus Selector/2 -> [fft1]  "<fft1>"
  0/2(sync_out) -> Terminator/1
  1/2(sync_out) -> Terminator1/1
  10/2(sync_out) -> Terminator2/1
  11/2(sync_out) -> Terminator3/1
  12/2(sync_out) -> Terminator4/1
  13/2(sync_out) -> Terminator5/1
  14/2(sync_out) -> Terminator6/1
  15/2(sync_out) -> Terminator7/1
  2/2(sync_out) -> Terminator8/1
  3/2(sync_out) -> Terminator9/1
  4/2(sync_out) -> Terminator10/1
  5/2(sync_out) -> Delay1/1  "sync"
  6/2(sync_out) -> Terminator12/1
  8/2(sync_out) -> Terminator13/1
  9/2(sync_out) -> Terminator14/1
  7/2(sync_out) -> Terminator11/1
  Concat/1 -> Delay2/1  "data"
  Delay1/1 -> Bus Creator/2  "sync"
  [eq0] -> eq_snap/1(In1)
  [eq1] -> eq_snap/2(In2)
  [eq2] -> eq_snap/3(In3)
  [eq3] -> eq_snap/4(In4)

== system roach2_fengine/eth ==
  note: (old)
      | IP and MAC address assignments for ports connected to switch
      | eth0: IP=10.10.10.(32+FID)  MAC=02:02:0A:0A:0A:(20+FID)
      | eth1: IP=10.10.10.(40+FID)  MAC=02:02:0A:0A:0A:(28+FID)
      | eth2: IP=10.10.10.(48+FID)  MAC=02:02:0A:0A:0A:(30+FID)
      | eth3: IP=10.10.10.(56+FID)  MAC=02:02:0A:0A:0A:(38+FID)
  note: ********old********
      | IP and MAC address assignments for ports connected to GPU X engines
      | eth0: IP=192.168.2.(1+FID)  MAC=02:02:C0:A8:02:(1+FID)
      | eth1: IP=192.168.3.(1+FID)  MAC=02:02:C0:A8:03:(1+FID)
      | eth2: IP=192.168.4.(1+FID)  MAC=02:02:C0:A8:04:(1+FID)
      | eth3: IP=192.168.5.(1+FID)  MAC=02:02:C0:A8:05:(1+FID)
  note: ctrl[8] = cnt_rst
      | ctrl[5] = gbe_gpu_rst
      | ctrl[4] = gbe_sw_rdst
      | ctrl[1] = gbe_gpu_en
      | ctrl[0] = gbe_sw_en
  note: (present)
      | IP and MAC address assignments for ports connected to switch
      | eth0: IP=10.10.10.(FID)  MAC=02:02:0A:0A:0A:(FID)
      | eth1: IP=10.10.10.(8+FID)  MAC=02:02:0A:0A:0A:(8+FID)
      | eth2: IP=10.10.10.(16+FID)  MAC=02:02:0A:0A:0A:(10+FID)
      | eth3: IP=10.10.10.(24+FID)  MAC=02:02:0A:0A:0A:(18+FID)
  In1 : Inport
  0 : Subsystem
  1 : Subsystem
  2 : Subsystem
  3 : Subsystem
  BitBasher : xbsIndex_r4/BitBasher  bitexpr=|  display_expr=off  sr_1=1  arith_type1=Unsigned  bin_pt1=0  sr_2=2  arith_type2=Unsigned  bin_pt2=0  sr_3=3  arith_type3=Unsigned  bin_pt3=0  sr_4=4  arith_type4=Unsigned  bin_pt4=0  dbl_ovrd=off  block_type=bitbasher
      | <!DOCTYPE HTML PUBLIC "-//W3C//DTD HTML 4.0//EN" "http://www.w3.org/TR/REC-html40/strict.dtd">
      | <html><head><meta name="qrichtext" content="1" /><style type="text/css">
      | p, li { white-space: pre-wrap; }
      | </style></head><body style=" font-family:'Sans'; font-size:10pt; font-weight:400; font-style:normal;">
      | <p style=" margin-top:12px; margin-bottom:12px; margin-left:0px; margin-right:0px; -qt-block-indent:0; text-indent:0px;"><span style=" font-family:'MS Shell Dlg'; font-size:8pt;">gpurst={ctrl[5]}<br />swrst={ctrl[4]}<br />gpuen={ctrl[1]}<br />swen={ctrl[0]}</span></p></body></html>
  BitBasher1 : xbsIndex_r4/BitBasher  bitexpr=|  display_expr=off  sr_1=1  arith_type1=Unsigned  bin_pt1=0  sr_2=2  arith_type2=Unsigned  bin_pt2=0  sr_3=3  arith_type3=Unsigned  bin_pt3=0  sr_4=4  arith_type4=Unsigned  bin_pt4=0  dbl_ovrd=off  block_type=bitbasher
      | <!DOCTYPE HTML PUBLIC "-//W3C//DTD HTML 4.0//EN" "http://www.w3.org/TR/REC-html40/strict.dtd">
      | <html><head><meta name="qrichtext" content="1" /><style type="text/css">
      | p, li { white-space: pre-wrap; }
      | </style></head><body style=" font-family:'Sans'; font-size:10pt; font-weight:400; font-style:normal;">
      | <p style=" margin-top:12px; margin-bottom:12px; margin-left:0px; margin-right:0px; -qt-block-indent:0; text-indent:0px;"><span style=" font-family:'MS Shell Dlg'; font-size:8pt;">cntrst={ctrl[8]}<br /></span></p></body></html>
  Bus Selector2 : BusSelector  OutputSignals=chan_0_255,chan_256_511,chan_512_767,chan_768_1023
  Constant1 : Constant  Value=0
  Constant3 : xbsIndex_r4/Constant  const=0  gui_display_data_type=Fixed-point  arith_type=Unsigned  n_bits=2  bin_pt=0  preci_type=Single  exp_width=8  frac_width=24  explicit_period=on  period=1  equ=P=C  opselect=C  inp2=PCIN>>17  opr=+  inp1=P  carry=CIN  dbl_ovrd=off  block_type=constant
  Constant5 : xbsIndex_r4/Constant  const=1  gui_display_data_type=Fixed-point  arith_type=Unsigned  n_bits=2  bin_pt=0  preci_type=Single  exp_width=8  frac_width=24  explicit_period=on  period=1  equ=P=C  opselect=C  inp2=PCIN>>17  opr=+  inp1=P  carry=CIN  dbl_ovrd=off  block_type=constant
  Constant7 : xbsIndex_r4/Constant  const=2  gui_display_data_type=Fixed-point  arith_type=Unsigned  n_bits=2  bin_pt=0  preci_type=Single  exp_width=8  frac_width=24  explicit_period=on  period=1  equ=P=C  opselect=C  inp2=PCIN>>17  opr=+  inp1=P  carry=CIN  dbl_ovrd=off  block_type=constant
  Constant8 : xbsIndex_r4/Constant  const=3  gui_display_data_type=Fixed-point  arith_type=Unsigned  n_bits=2  bin_pt=0  preci_type=Single  exp_width=8  frac_width=24  explicit_period=on  period=1  equ=P=C  opselect=C  inp2=PCIN>>17  opr=+  inp1=P  carry=CIN  dbl_ovrd=off  block_type=constant
  Convert{1..5} (5 alike) : xbsIndex_r4/Convert  gui_display_data_type=Boolean  arith_type=Boolean  n_bits=32  bin_pt=0  float_type=Single  exp_bits=8  fraction_bits=24  quantization=Truncate  overflow=Wrap  en=off  latency=0  dbl_ovrd=off  pipeline=off  block_type=convert
      settings: block_version=11.4
  Delay2 : xbsIndex_r4/Delay  rst=off  infoeditControl=Selection of Reset will increase slice count due to use of real FFs and instead of SRLs  en=off  latency=2  dbl_ovrd=off  reg_retiming=on  block_type=delay
  ctrl : Subsystem  Tag=xps:sw_reg  AncestorBlock=xps_library/software register  mask{numios=1, io_dir=From Processor, io_delay=0, sample_period=1, name1=reg, bitwidth1=32, arith_type1=Unsigned, bin_pt1=0}
      mask prompts: numios "Num I/Os"; io_dir "I/O direction"; io_delay "I/O delay"; sample_period "Sample period"; name1 "Name"; bitwidth1 "Bitwidth"; arith_type1 "Type"; bin_pt1 "Binary pt"
      mask init:
      | swreg_init(gcb);
      mask StyleString: edit,popup(To Processor|From Processor),edit,edit,edit,edit,popup(Unsigned|Signed  (2's comp)|Boolean),edit
      mask CallbackString:
      | t = timer('StartDelay', 0.1); t.TimerFcn = {@swreg_cb, gcb}; start(t);|a = get_param(gcb, 'MaskEnables');
      | if strcmp(get_param(gcb, 'io_dir'), 'To Processor'),
      | a(4) = {'off'};
      | else
      | a(4) = {'on'};
      | end
      | set_param(gcb, 'MaskEnables', a);
      | |||||a = get_param(gcb, 'MaskEnables');
      | if strcmp(get_param(gcb, 'arith_type1'), 'Boolean'),
      | set_param(gcb, 'bitwidth1', '1');
      | set_param(gcb, 'bin_pt1', '0');
      | a(6) = {'off'};
      | a(8) = {'off'};
      | else
      | a(6) = {'on'};
      | a(8) = {'on'};
      | end
      | set_param(gcb, 'MaskEnables', a);
      | |
      mask TabNameString: Setup,Setup,Setup,Setup,IO1,IO1,IO1,IO1
  tag scope: cnt_rst=global, gbe_gpu_en=global, gbe_gpu_rst=global, gbe_sw_en=global, gbe_sw_rst=global
  wires:
  In1/1 -> Bus Selector2/1
  [chan_256_511] -> 1/1(data)
  Bus Selector2/1 -> [chan_0_255]  "<chan_0_255>"
  Bus Selector2/2 -> [chan_256_511]  "<chan_256_511>"
  Bus Selector2/3 -> [chan_512_767]  "<chan_512_767>"
  Bus Selector2/4 -> [chan_768_1023]  "<chan_768_1023>"
  [chan_0_255] -> 0/1(data)
  [chan_768_1023] -> 3/1(data)
  [chan_512_767] -> 2/1(data)
  Constant1/1 -> ctrl/1(sim_reg)
  BitBasher/1 -> Convert2/1
  BitBasher/2 -> Convert3/1
  BitBasher/3 -> Convert4/1
  BitBasher/4 -> Convert5/1
  BitBasher1/1 -> Convert1/1
  Convert2/1 -> [gbe_gpu_rst]
  Convert1/1 -> [cnt_rst]
  Convert3/1 -> [gbe_sw_rst]
  Convert4/1 -> [gbe_gpu_en]
  ctrl/1(in_reg) -> Delay2/1
  Delay2/1 -> BitBasher/1, BitBasher1/1
  Constant3/1 -> 0/2(sw_base)
  Constant7/1 -> 2/2(sw_base)
  Constant8/1 -> 3/2(sw_base)
  Constant5/1 -> 1/2(sw_base)
  Convert5/1 -> [gbe_sw_en]

== system roach2_fengine/fft_shift ==
  sim_reg : Inport
  io_delay : xbsIndex_r4/Delay  rst=off  infoeditControl=Selection of Reset will increase slice count due to use of real FFs and instead of SRLs  en=off  latency=0  dbl_ovrd=off  reg_retiming=on  block_type=delay
  reint1 : xbsIndex_r4/Reinterpret  force_arith_type=on  arith_type=Unsigned  force_bin_pt=on  bin_pt=0  block_type=reinterpret
  roach2_fengine_fft_shift_user_data_out : xbsIndex_r4/Gateway In  gui_display_data_type=Fixed-point  arith_type=Unsigned  n_bits=32  bin_pt=0  preci_type=Single  exp_width=8  frac_width=24  quantization="Round  (unbiased: +/- Inf)"  overflow=Saturate  period=1  dbl_ovrd=off  timing_constraint=None  locs_specified=off  LOCs={}  UseAsADC=off  ADCChannel='1'  inherit_from_input=off  hdl_port=on  block_type=gatewayin
  slice_reg : xbsIndex_r4/Slice  nbits=32  boolean_output=off  mode=Lower Bit Location + Width  bit1=0  base1=MSB of Input  bit0=0  base0=LSB of Input  dbl_ovrd=off  block_type=slice
  in_reg : Outport
  wires:
  roach2_fengine_fft_shift_user_data_out/1 -> io_delay/1
  sim_reg/1 -> roach2_fengine_fft_shift_user_data_out/1
  io_delay/1 -> slice_reg/1
  slice_reg/1 -> reint1/1
  reint1/1 -> in_reg/1

== system roach2_fengine/fft_shift_slicer ==
  In1 : Inport
  Convert : xbsIndex_r4/Convert  gui_display_data_type=Fixed-point  arith_type=Unsigned  n_bits=16  bin_pt=0  float_type=Single  exp_bits=8  fraction_bits=24  quantization=Truncate  overflow=Wrap  en=off  latency=0  dbl_ovrd=off  pipeline=off  block_type=convert
  Delay8 : xbsIndex_r4/Delay  rst=off  infoeditControl=Selection of Reset will increase slice count due to use of real FFs and instead of SRLs  en=off  latency=2  dbl_ovrd=off  reg_retiming=on  block_type=delay
      settings: xl_area=[0, 0, 0, 0, 0, 0, 0]
  b10_b0 : xbsIndex_r4/Slice  nbits=11  boolean_output=off  mode=Lower Bit Location + Width  bit1=0  base1=MSB of Input  bit0=0  base0=LSB of Input  dbl_ovrd=off  block_type=slice
      settings: block_version=10.1.3
  b12_b11 : xbsIndex_r4/Slice  nbits=2  boolean_output=off  mode=Lower Bit Location + Width  bit1=0  base1=MSB of Input  bit0=11  base0=LSB of Input  dbl_ovrd=off  block_type=slice
      settings: block_version=10.1.3
  tag scope: fft_preshift=global, fft_shift=global
  wires:
  Delay8/1 -> b10_b0/1, b12_b11/1
  b10_b0/1 -> Convert/1
  b12_b11/1 -> [fft_preshift]
  In1/1 -> Delay8/1
  Convert/1 -> [fft_shift]

== system roach2_fengine/fid ==
  sim_reg : Inport
  io_delay : xbsIndex_r4/Delay  rst=off  infoeditControl=Selection of Reset will increase slice count due to use of real FFs and instead of SRLs  en=off  latency=0  dbl_ovrd=off  reg_retiming=on  block_type=delay
  reint1 : xbsIndex_r4/Reinterpret  force_arith_type=on  arith_type=Unsigned  force_bin_pt=on  bin_pt=0  block_type=reinterpret
  roach2_fengine_fid_user_data_out : xbsIndex_r4/Gateway In  gui_display_data_type=Fixed-point  arith_type=Unsigned  n_bits=32  bin_pt=0  preci_type=Single  exp_width=8  frac_width=24  quantization="Round  (unbiased: +/- Inf)"  overflow=Saturate  period=1  dbl_ovrd=off  timing_constraint=None  locs_specified=off  LOCs={}  UseAsADC=off  ADCChannel='1'  inherit_from_input=off  hdl_port=on  block_type=gatewayin
  slice_reg : xbsIndex_r4/Slice  nbits=32  boolean_output=off  mode=Lower Bit Location + Width  bit1=0  base1=MSB of Input  bit0=0  base0=LSB of Input  dbl_ovrd=off  block_type=slice
  in_reg : Outport
  wires:
  roach2_fengine_fid_user_data_out/1 -> io_delay/1
  sim_reg/1 -> roach2_fengine_fid_user_data_out/1
  io_delay/1 -> slice_reg/1
  slice_reg/1 -> reint1/1
  reint1/1 -> in_reg/1

== system roach2_fengine/fid_slicer ==
  In1 : Inport
  Delay8 : xbsIndex_r4/Delay  rst=off  infoeditControl=Selection of Reset will increase slice count due to use of real FFs and instead of SRLs  en=off  latency=2  dbl_ovrd=off  reg_retiming=on  block_type=delay
      settings: xl_area=[0, 0, 0, 0, 0, 0, 0]
  b2_b0 : xbsIndex_r4/Slice  nbits=3  boolean_output=off  mode=Lower Bit Location + Width  bit1=0  base1=MSB of Input  bit0=0  base0=LSB of Input  dbl_ovrd=off  block_type=slice
      settings: block_version=10.1.3
  tag scope: FID=global
  wires:
  Delay8/1 -> b2_b0/1
  b2_b0/1 -> [FID]
  In1/1 -> Delay8/1
